simulink model "AcasXuClosedLoop_all_comp2017b"  (Simulink 9.0)
settings: Solver=VariableStepAuto  StartTime=0.0  StopTime=20  RelTol=1e-3
libraries: neural x14040, simulink x2
(part 18 of 534)

== system AcasXuClosedLoop_all_comp2017b/networks/Networks_m_4/AcasXu_2_4/Layer 3 ==
  "a{2} " : Inport  PortDimensions=50
      mask Display: e = 0.06;c1 = [120 230 180]/255;c2 = ([120 230 180]+0)/(256+128);c3 = ([120 230 180]+128)/(256+128);patch([0 1 1 0],[0 0 1 1],c1);patch([0 1 1 1-e 1-e 0+e],[0 0 1 1-e 0+e 0+e],c2);patch([0 0 1 1-e 0+e 0+e],[0 1 1 1-e 1-e 0+e],c3);plot([0 1 1 0 0],[0 0 1 1 0]);
  Delays 1 : Subsystem
      mask Display: e = 0.06;c1 = [120 150 230]/255;c2 = ([120 150 230]+0)/383;c3 = ([120 150 230]+128)/383;patch([0 1 1 0],[0 0 1 1],c1);patch([0 1 1 1-e 1-e 0+e],[0 0 1 1-e 0+e 0+e],c2);patch([0 0 1 1-e 0+e 0+e],[0 1 1 1-e 1-e 0+e],c3);plot([0 1 1 0 0],[0 0 1 1 0]);disp('0');
  "LW{3,2}" : Subsystem
      mask Display: e = 0.06;c1 = [120 150 230]/255;c2 = ([120 150 230]+0)/383;c3 = ([120 150 230]+128)/383;patch([0 1 1 0],[0 0 1 1],c1);patch([0 1 1 1-e 1-e 0+e],[0 0 1 1-e 0+e 0+e],c2);patch([0 0 1 1-e 0+e 0+e],[0 1 1 1-e 1-e 0+e],c3);plot([0 1 1 0 0],[0 0 1 1 0]);disp('W');
  "b{3}" : Constant  Value=[-0.56579800000000002313527147634886205196380615234375;0.6863989999999999813695694683701731264591217041015625;0.32173499999999999321431687349104322493076324462890625;0.07862260000000000081943341001533553935587406158447265625;-0.19624099999999999877076106713502667844295501708984375;0.122257000000000004558131649901042692363262176513671875;-1.1890300000000000313349346470204181969165802001953125;-0.8600090000000000234336994253681041300296783447265625;-1.40829000000000004177991286269389092922210693359375;-2.094539999999999846380660528666339814662933349609375;-1.008070000000000021600499167107045650482177734375;-0.342619999999999980122566967111197300255298614501953125;-0.2805210000000000203357330974540673196315765380859375;0.1626039999999999985380583211735938675701618194580078125;-1.042990000000000083701934272539801895618438720703125;0.62873400000000001508482228018692694604396820068359375;0.50152900000000000257927013080916367471218109130859375;-0.60730899999999998772892695342306979000568389892578125;-3.88682000000000016370904631912708282470703125;-0.365016999999999980364151497269631363451480865478515625;-0.251825000000000021049828546892968006432056427001953125;0.2107259999999999966480146440517273731529712677001953125;-0.99212800000000000988364945442299358546733856201171875;-0.297250999999999987455368000155431218445301055908203125;0.380684999999999995612398606681381352245807647705078125;-0.8203549999999999453592636200482957065105438232421875;-0.034085999999999998244515353462702478282153606414794921875;-0.9310359999999999747188894616556353867053985595703125;-1.346950000000000091660012913052923977375030517578125;1.1735800000000000675726141707855276763439178466796875;0.55995399999999995177546452396200038492679595947265625;-0.1108179999999999998383515276145772077143192291259765625;1.505549999999999943867123874952085316181182861328125;-0.246101999999999987434051718082628212869167327880859375;-0.72938099999999994604849007373559288680553436279296875;-1.30217999999999989313437254168093204498291015625;-0.2433309999999999917452697673070360906422138214111328125;0.790510999999999963705477057374082505702972412109375;1.1992799999999999016608853708021342754364013671875;-0.63741700000000001136157834480400197207927703857421875;-0.016549899999999999333777367382936063222587108612060546875;-0.60409900000000005260147872832021676003932952880859375;-0.46064899999999997515232053046929650008678436279296875;1.1716200000000001057998133546789176762104034423828125;0.7013759999999999994457766661071218550205230712890625;-0.18702099999999999280220208675018511712551116943359375;-0.020953800000000001590994003208834328688681125640869140625;-0.885156999999999971606712279026396572589874267578125;0.501260999999999956600049699773080646991729736328125;-1.2725400000000000044764192352886311709880828857421875]
      mask Display: e = 0.06;c1 = [120 150 230]/255;c2 = ([120 150 230]+0)/383;c3 = ([120 150 230]+128)/383;patch([0 1 1 0],[0 0 1 1],c1);patch([0 1 1 1-e 1-e 0+e],[0 0 1 1-e 0+e 0+e],c2);patch([0 0 1 1-e 0+e 0+e],[0 1 1 1-e 1-e 0+e],c3);plot([0 1 1 0 0],[0 0 1 1 0]);disp('b');
  netsum : Sum  Inputs=2  SaturateOnIntegerOverflow=on  InputSameDT=on  OutDataTypeStr=Inherit: Same as first input
      mask Display: e = 0.06;c1 = [120 150 230]/255;c2 = ([120 150 230]+0)/383;c3 = ([120 150 230]+128)/383;patch([0 1 1 0],[0 0 1 1],c1);patch([0 1 1 1-e 1-e 0+e],[0 0 1 1-e 0+e 0+e],c2);patch([0 0 1 1-e 0+e 0+e],[0 1 1 1-e 1-e 0+e],c3);plot([0 1 1 0 0],[0 0 1 1 0]);disp('+');
  poslin : neural/Transfer Functions/poslin
      settings: SourceProductName=Deep Learning Toolbox  SourceProductBaseCode=NN
  "a{3}" : Outport  InitialOutput=[0;0;0;0;0;0;0;0;0;0;0;0;0;0;0;0;0;0;0;0;0;0;0;0;0;0;0;0;0;0;0;0;0;0;0;0;0;0;0;0;0;0;0;0;0;0;0;0;0;0]
      mask Display: e = 0.06;c1 = [120 230 180]/255;c2 = ([120 230 180]+0)/(256+128);c3 = ([120 230 180]+128)/(256+128);patch([0 1 1 0],[0 0 1 1],c1);patch([0 1 1 1-e 1-e 0+e],[0 0 1 1-e 0+e 0+e],c2);patch([0 0 1 1-e 0+e 0+e],[0 1 1 1-e 1-e 0+e],c3);plot([0 1 1 0 0],[0 0 1 1 0]);
  wires:
  poslin/1 -> "a{3}"/1
  netsum/1 -> poslin/1
  "b{3}"/1 -> netsum/2
  "LW{3,2}"/1("lz{3,2}") -> netsum/1
  Delays 1/1("pd{3,2}") -> "LW{3,2}"/1("ad{3,2}")
  "a{2} "/1 -> Delays 1/1("p{3}")

== system AcasXuClosedLoop_all_comp2017b/networks/Networks_m_4/AcasXu_2_4/Layer 4 ==
  "a{3} " : Inport  PortDimensions=50
      mask Display: e = 0.06;c1 = [120 230 180]/255;c2 = ([120 230 180]+0)/(256+128);c3 = ([120 230 180]+128)/(256+128);patch([0 1 1 0],[0 0 1 1],c1);patch([0 1 1 1-e 1-e 0+e],[0 0 1 1-e 0+e 0+e],c2);patch([0 0 1 1-e 0+e 0+e],[0 1 1 1-e 1-e 0+e],c3);plot([0 1 1 0 0],[0 0 1 1 0]);
  Delays 1 : Subsystem
      mask Display: e = 0.06;c1 = [120 150 230]/255;c2 = ([120 150 230]+0)/383;c3 = ([120 150 230]+128)/383;patch([0 1 1 0],[0 0 1 1],c1);patch([0 1 1 1-e 1-e 0+e],[0 0 1 1-e 0+e 0+e],c2);patch([0 0 1 1-e 0+e 0+e],[0 1 1 1-e 1-e 0+e],c3);plot([0 1 1 0 0],[0 0 1 1 0]);disp('0');
  "LW{4,3}" : Subsystem
      mask Display: e = 0.06;c1 = [120 150 230]/255;c2 = ([120 150 230]+0)/383;c3 = ([120 150 230]+128)/383;patch([0 1 1 0],[0 0 1 1],c1);patch([0 1 1 1-e 1-e 0+e],[0 0 1 1-e 0+e 0+e],c2);patch([0 0 1 1-e 0+e 0+e],[0 1 1 1-e 1-e 0+e],c3);plot([0 1 1 0 0],[0 0 1 1 0]);disp('W');
  "b{4}" : Constant  Value=[-0.0139097000000000005359712673680405714549124240875244140625;-0.07528880000000000294324564720227499492466449737548828125;2.075530000000000097060137704829685389995574951171875;0.100026000000000003797850922637735493481159210205078125;-0.69344799999999995332444768791901879012584686279296875;-1.270750000000000046185277824406512081623077392578125;-1.3205199999999999160849029067321680486202239990234375;-1.0355399999999999050004362288746051490306854248046875;-1.220310000000000005826450433232821524143218994140625;-1.7545900000000000940048039410612545907497406005859375;-0.12118299999999999905231362617996637709438800811767578125;-2.16260999999999992127186487778089940547943115234375;-0.14306900000000000172661884789704345166683197021484375;-0.0989629999999999954152229975079535506665706634521484375;0.61942799999999997861976908097858540713787078857421875;-2.328440000000000065227823142777197062969207763671875;-0.935335999999999945231365927611477673053741455078125;-0.56300300000000003119993152722599916160106658935546875;-3.3324400000000000687805368215776979923248291015625;-1.3442600000000000104449782156734727323055267333984375;-0.0438336000000000003407052417969680391252040863037109375;0.54565099999999999713651277488679625093936920166015625;-1.4457299999999999595701183352502994239330291748046875;-0.284542000000000017134738072854815982282161712646484375;-0.56924200000000002575006874394603073596954345703125;-0.5276710000000000011510792319313623011112213134765625;0.331886999999999987576160265234648250043392181396484375;-1.167240000000000055280224842135794460773468017578125;0.59963500000000002909672502937610261142253875732421875;-1.1537599999999998967581404940574429929256439208984375;0.67512899999999997913135985072585754096508026123046875;0.046315399999999999625099889044577139429748058319091796875;-0.7551639999999999464108668689732439815998077392578125;0.90475099999999997191224565540323965251445770263671875;-1.098360000000000002984279490192420780658721923828125;0.490709000000000006291855925155687145888805389404296875;-1.830340000000000078017592386459000408649444580078125;-0.330212000000000005517364343177177943289279937744140625;-0.76566400000000001124789150708238594233989715576171875;1.3308999999999999719335619374760426580905914306640625;-0.0499606999999999967787545074315858073532581329345703125;-0.033070599999999998608313234171873773448169231414794921875;0.943799999999999972288833305356092751026153564453125;-1.420619999999999993889332472463138401508331298828125;1.1566799999999999304378661690861918032169342041015625;0.4695300000000000029132252166164107620716094970703125;-0.12093800000000000383071352416664012707769870758056640625;-1.2574799999999999311484089048462919890880584716796875;0.2654279999999999972715158946812152862548828125;-1.13037000000000009691802915767766535282135009765625]
      mask Display: e = 0.06;c1 = [120 150 230]/255;c2 = ([120 150 230]+0)/383;c3 = ([120 150 230]+128)/383;patch([0 1 1 0],[0 0 1 1],c1);patch([0 1 1 1-e 1-e 0+e],[0 0 1 1-e 0+e 0+e],c2);patch([0 0 1 1-e 0+e 0+e],[0 1 1 1-e 1-e 0+e],c3);plot([0 1 1 0 0],[0 0 1 1 0]);disp('b');
  netsum : Sum  Inputs=2  SaturateOnIntegerOverflow=on  InputSameDT=on  OutDataTypeStr=Inherit: Same as first input
      mask Display: e = 0.06;c1 = [120 150 230]/255;c2 = ([120 150 230]+0)/383;c3 = ([120 150 230]+128)/383;patch([0 1 1 0],[0 0 1 1],c1);patch([0 1 1 1-e 1-e 0+e],[0 0 1 1-e 0+e 0+e],c2);patch([0 0 1 1-e 0+e 0+e],[0 1 1 1-e 1-e 0+e],c3);plot([0 1 1 0 0],[0 0 1 1 0]);disp('+');
  poslin : neural/Transfer Functions/poslin
      settings: SourceProductName=Deep Learning Toolbox  SourceProductBaseCode=NN
  "a{4}" : Outport  InitialOutput=[0;0;0;0;0;0;0;0;0;0;0;0;0;0;0;0;0;0;0;0;0;0;0;0;0;0;0;0;0;0;0;0;0;0;0;0;0;0;0;0;0;0;0;0;0;0;0;0;0;0]
      mask Display: e = 0.06;c1 = [120 230 180]/255;c2 = ([120 230 180]+0)/(256+128);c3 = ([120 230 180]+128)/(256+128);patch([0 1 1 0],[0 0 1 1],c1);patch([0 1 1 1-e 1-e 0+e],[0 0 1 1-e 0+e 0+e],c2);patch([0 0 1 1-e 0+e 0+e],[0 1 1 1-e 1-e 0+e],c3);plot([0 1 1 0 0],[0 0 1 1 0]);
  wires:
  poslin/1 -> "a{4}"/1
  netsum/1 -> poslin/1
  "b{4}"/1 -> netsum/2
  "LW{4,3}"/1("lz{4,3}") -> netsum/1
  Delays 1/1("pd{4,3}") -> "LW{4,3}"/1("ad{4,3}")
  "a{3} "/1 -> Delays 1/1("p{4}")

== system AcasXuClosedLoop_all_comp2017b/networks/Networks_m_4/AcasXu_2_4/Layer 5 ==
  "a{4} " : Inport  PortDimensions=50
      mask Display: e = 0.06;c1 = [120 230 180]/255;c2 = ([120 230 180]+0)/(256+128);c3 = ([120 230 180]+128)/(256+128);patch([0 1 1 0],[0 0 1 1],c1);patch([0 1 1 1-e 1-e 0+e],[0 0 1 1-e 0+e 0+e],c2);patch([0 0 1 1-e 0+e 0+e],[0 1 1 1-e 1-e 0+e],c3);plot([0 1 1 0 0],[0 0 1 1 0]);
  Delays 1 : Subsystem
      mask Display: e = 0.06;c1 = [120 150 230]/255;c2 = ([120 150 230]+0)/383;c3 = ([120 150 230]+128)/383;patch([0 1 1 0],[0 0 1 1],c1);patch([0 1 1 1-e 1-e 0+e],[0 0 1 1-e 0+e 0+e],c2);patch([0 0 1 1-e 0+e 0+e],[0 1 1 1-e 1-e 0+e],c3);plot([0 1 1 0 0],[0 0 1 1 0]);disp('0');
  "LW{5,4}" : Subsystem
      mask Display: e = 0.06;c1 = [120 150 230]/255;c2 = ([120 150 230]+0)/383;c3 = ([120 150 230]+128)/383;patch([0 1 1 0],[0 0 1 1],c1);patch([0 1 1 1-e 1-e 0+e],[0 0 1 1-e 0+e 0+e],c2);patch([0 0 1 1-e 0+e 0+e],[0 1 1 1-e 1-e 0+e],c3);plot([0 1 1 0 0],[0 0 1 1 0]);disp('W');
  "b{5}" : Constant  Value=[-0.10940999999999999336974809693856514059007167816162109375;-4.41561000000000003495870259939692914485931396484375;1.2665800000000000391509047403815202414989471435546875;-0.457340000000000024282797994601423852145671844482421875;2.1883499999999997953636921010911464691162109375;-0.366215000000000012736478538499795831739902496337890625;0.365192000000000016601831021034740842878818511962890625;-1.48285000000000000142108547152020037174224853515625;0.1785130000000000050075499302693060599267482757568359375;0.09404089999999999671675965373651706613600254058837890625;0.5114760000000000417230694438330829143524169921875;-1.9412700000000000510880227011512033641338348388671875;-0.70229799999999997783817207164247520267963409423828125;-0.141270000000000006679101716144941747188568115234375;1.062689999999999912461134954355657100677490234375;0.52761899999999994914645640164962969720363616943359375;0.78126799999999996249044897922431118786334991455078125;-1.9535199999999999231903302643331699073314666748046875;-2.3968199999999999505462255910970270633697509765625;-0.78913599999999994860644392247195355594158172607421875;0.2007210000000000105213615597676835022866725921630859375;-0.77595599999999997908872728658025152981281280517578125;0.717330000000000023163693185779266059398651123046875;-1.6638800000000000256505927609396167099475860595703125;1.08101000000000002643218977027572691440582275390625;-0.0638539999999999940971662226729677058756351470947265625;1.567190000000000082991391536779701709747314453125;0.041038499999999998590904937145751318894326686859130859375;1.7739799999999998902922015986405313014984130859375;-0.0660338000000000036049385698788682930171489715576171875;0.495254000000000027537083724382682703435420989990234375;-2.619190000000000129176669361186213791370391845703125;1.66504999999999991899812812334857881069183349609375;-0.67660299999999995446131606513517908751964569091796875;0.41489100000000000978417347141657955944538116455078125;-1.2167600000000000637356833976809866726398468017578125;1.1150599999999999401012473754235543310642242431640625;0.9283310000000000172803993336856365203857421875;0.2296999999999999875210932032132404856383800506591796875;0.053061999999999998056665617696125991642475128173828125;2.1252300000000001745092959026806056499481201171875;-1.03211999999999992638777257525362074375152587890625;0.6291889999999999982804865794605575501918792724609375;-3.50731999999999999317878973670303821563720703125;-0.507355000000000000426325641456060111522674560546875;0.372373000000000009546141654936945997178554534912109375;0.9320180000000000131166189021314494311809539794921875;-0.50340200000000001612221467439667321741580963134765625;-1.4255400000000000293454149868921376764774322509765625;0.883040999999999964842345434590242803096771240234375]
      mask Display: e = 0.06;c1 = [120 150 230]/255;c2 = ([120 150 230]+0)/383;c3 = ([120 150 230]+128)/383;patch([0 1 1 0],[0 0 1 1],c1);patch([0 1 1 1-e 1-e 0+e],[0 0 1 1-e 0+e 0+e],c2);patch([0 0 1 1-e 0+e 0+e],[0 1 1 1-e 1-e 0+e],c3);plot([0 1 1 0 0],[0 0 1 1 0]);disp('b');
  netsum : Sum  Inputs=2  SaturateOnIntegerOverflow=on  InputSameDT=on  OutDataTypeStr=Inherit: Same as first input
      mask Display: e = 0.06;c1 = [120 150 230]/255;c2 = ([120 150 230]+0)/383;c3 = ([120 150 230]+128)/383;patch([0 1 1 0],[0 0 1 1],c1);patch([0 1 1 1-e 1-e 0+e],[0 0 1 1-e 0+e 0+e],c2);patch([0 0 1 1-e 0+e 0+e],[0 1 1 1-e 1-e 0+e],c3);plot([0 1 1 0 0],[0 0 1 1 0]);disp('+');
  poslin : neural/Transfer Functions/poslin
      settings: SourceProductName=Deep Learning Toolbox  SourceProductBaseCode=NN
  "a{5}" : Outport  InitialOutput=[0;0;0;0;0;0;0;0;0;0;0;0;0;0;0;0;0;0;0;0;0;0;0;0;0;0;0;0;0;0;0;0;0;0;0;0;0;0;0;0;0;0;0;0;0;0;0;0;0;0]
      mask Display: e = 0.06;c1 = [120 230 180]/255;c2 = ([120 230 180]+0)/(256+128);c3 = ([120 230 180]+128)/(256+128);patch([0 1 1 0],[0 0 1 1],c1);patch([0 1 1 1-e 1-e 0+e],[0 0 1 1-e 0+e 0+e],c2);patch([0 0 1 1-e 0+e 0+e],[0 1 1 1-e 1-e 0+e],c3);plot([0 1 1 0 0],[0 0 1 1 0]);
  wires:
  poslin/1 -> "a{5}"/1
  netsum/1 -> poslin/1
  "b{5}"/1 -> netsum/2
  "LW{5,4}"/1("lz{5,4}") -> netsum/1
  Delays 1/1("pd{5,4}") -> "LW{5,4}"/1("ad{5,4}")
  "a{4} "/1 -> Delays 1/1("p{5}")

== system AcasXuClosedLoop_all_comp2017b/networks/Networks_m_4/AcasXu_2_4/Layer 6 ==
  "a{5} " : Inport  PortDimensions=50
      mask Display: e = 0.06;c1 = [120 230 180]/255;c2 = ([120 230 180]+0)/(256+128);c3 = ([120 230 180]+128)/(256+128);patch([0 1 1 0],[0 0 1 1],c1);patch([0 1 1 1-e 1-e 0+e],[0 0 1 1-e 0+e 0+e],c2);patch([0 0 1 1-e 0+e 0+e],[0 1 1 1-e 1-e 0+e],c3);plot([0 1 1 0 0],[0 0 1 1 0]);
  Delays 1 : Subsystem
      mask Display: e = 0.06;c1 = [120 150 230]/255;c2 = ([120 150 230]+0)/383;c3 = ([120 150 230]+128)/383;patch([0 1 1 0],[0 0 1 1],c1);patch([0 1 1 1-e 1-e 0+e],[0 0 1 1-e 0+e 0+e],c2);patch([0 0 1 1-e 0+e 0+e],[0 1 1 1-e 1-e 0+e],c3);plot([0 1 1 0 0],[0 0 1 1 0]);disp('0');
  "LW{6,5}" : Subsystem
      mask Display: e = 0.06;c1 = [120 150 230]/255;c2 = ([120 150 230]+0)/383;c3 = ([120 150 230]+128)/383;patch([0 1 1 0],[0 0 1 1],c1);patch([0 1 1 1-e 1-e 0+e],[0 0 1 1-e 0+e 0+e],c2);patch([0 0 1 1-e 0+e 0+e],[0 1 1 1-e 1-e 0+e],c3);plot([0 1 1 0 0],[0 0 1 1 0]);disp('W');
  "b{6}" : Constant  Value=[0.2346999999999999919619853017138666473329067230224609375;-1.9369099999999999095479097377392463386058807373046875;-0.475692999999999976967757220336352474987506866455078125;-0.384604000000000001424638185199000872671604156494140625;-0.023999300000000001187316911455127410590648651123046875;0.126871000000000011542766742422827519476413726806640625;0.132994000000000001104893954106955789029598236083984375;-1.081090000000000106439301816863007843494415283203125;0.060183500000000000884625706021324731409549713134765625;-0.154803999999999997161381770638399757444858551025390625;-0.71192200000000005477573949974612332880496978759765625;-0.60855899999999996108357436241931281983852386474609375;0.05336849999999999927258187426559743471443653106689453125;0.055166500000000000258904009342586505226790904998779296875;0.449330999999999980420994916130439378321170806884765625;-0.33911999999999997701394249816075898706912994384765625;-0.0885939000000000032031266528065316379070281982421875;-2.646549999999999958077978590154089033603668212890625;-0.194010999999999989018562018827651627361774444580078125;0.414953999999999989523047361217322759330272674560546875;0.492707999999999979312548248344683088362216949462890625;-0.10349999999999999478195178426176426000893115997314453125;0.38083299999999997709210219909437000751495361328125;0.09757749999999999757083202212015748955309391021728515625;-0.315176999999999984947152142922277562320232391357421875;0.301354999999999983995024877003743313252925872802734375;-0.9592460000000000430731006417772732675075531005859375;-0.4030110000000000081143980423803441226482391357421875;-0.2432850000000000012523315717771765775978565216064453125;0.464538999999999979717557607727940194308757781982421875;0.244925000000000003819167204710538499057292938232421875;-0.6850840000000000262758703684085048735141754150390625;-0.34977999999999997982058630441315472126007080078125;-0.8555509999999999504183278986602090299129486083984375;-2.034009999999999873665501581854186952114105224609375;-0.36635200000000001097077984013594686985015869140625;0.014788400000000000156230584025252028368413448333740234375;0.319072999999999995512922623674967326223850250244140625;0.426536999999999999477751089216326363384723663330078125;0.262087000000000014399148540178430266678333282470703125;-0.1453480000000000049720227934813010506331920623779296875;-0.7604319999999999968309793985099531710147857666015625;-2.73768000000000011340262062731198966503143310546875;0.113178000000000000824229573481716215610504150390625;-0.054530900000000000205080397108758916147053241729736328125;0.1973979999999999901394431844892096705734729766845703125;-3.407529999999999947846163195208646357059478759765625;-0.05762799999999999867039690570891252718865871429443359375;-0.7910120000000000484163820146932266652584075927734375;0.7156569999999999875939238336286507546901702880859375]
      mask Display: e = 0.06;c1 = [120 150 230]/255;c2 = ([120 150 230]+0)/383;c3 = ([120 150 230]+128)/383;patch([0 1 1 0],[0 0 1 1],c1);patch([0 1 1 1-e 1-e 0+e],[0 0 1 1-e 0+e 0+e],c2);patch([0 0 1 1-e 0+e 0+e],[0 1 1 1-e 1-e 0+e],c3);plot([0 1 1 0 0],[0 0 1 1 0]);disp('b');
  netsum : Sum  Inputs=2  SaturateOnIntegerOverflow=on  InputSameDT=on  OutDataTypeStr=Inherit: Same as first input
      mask Display: e = 0.06;c1 = [120 150 230]/255;c2 = ([120 150 230]+0)/383;c3 = ([120 150 230]+128)/383;patch([0 1 1 0],[0 0 1 1],c1);patch([0 1 1 1-e 1-e 0+e],[0 0 1 1-e 0+e 0+e],c2);patch([0 0 1 1-e 0+e 0+e],[0 1 1 1-e 1-e 0+e],c3);plot([0 1 1 0 0],[0 0 1 1 0]);disp('+');
  poslin : neural/Transfer Functions/poslin
      settings: SourceProductName=Deep Learning Toolbox  SourceProductBaseCode=NN
  "a{6}" : Outport  InitialOutput=[0;0;0;0;0;0;0;0;0;0;0;0;0;0;0;0;0;0;0;0;0;0;0;0;0;0;0;0;0;0;0;0;0;0;0;0;0;0;0;0;0;0;0;0;0;0;0;0;0;0]
      mask Display: e = 0.06;c1 = [120 230 180]/255;c2 = ([120 230 180]+0)/(256+128);c3 = ([120 230 180]+128)/(256+128);patch([0 1 1 0],[0 0 1 1],c1);patch([0 1 1 1-e 1-e 0+e],[0 0 1 1-e 0+e 0+e],c2);patch([0 0 1 1-e 0+e 0+e],[0 1 1 1-e 1-e 0+e],c3);plot([0 1 1 0 0],[0 0 1 1 0]);
  wires:
  poslin/1 -> "a{6}"/1
  netsum/1 -> poslin/1
  "b{6}"/1 -> netsum/2
  "LW{6,5}"/1("lz{6,5}") -> netsum/1
  Delays 1/1("pd{6,5}") -> "LW{6,5}"/1("ad{6,5}")
  "a{5} "/1 -> Delays 1/1("p{6}")

== system AcasXuClosedLoop_all_comp2017b/networks/Networks_m_4/AcasXu_2_4/Layer 7 ==
  "a{6} " : Inport  PortDimensions=50
      mask Display: e = 0.06;c1 = [120 230 180]/255;c2 = ([120 230 180]+0)/(256+128);c3 = ([120 230 180]+128)/(256+128);patch([0 1 1 0],[0 0 1 1],c1);patch([0 1 1 1-e 1-e 0+e],[0 0 1 1-e 0+e 0+e],c2);patch([0 0 1 1-e 0+e 0+e],[0 1 1 1-e 1-e 0+e],c3);plot([0 1 1 0 0],[0 0 1 1 0]);
  Delays 1 : Subsystem
      mask Display: e = 0.06;c1 = [120 150 230]/255;c2 = ([120 150 230]+0)/383;c3 = ([120 150 230]+128)/383;patch([0 1 1 0],[0 0 1 1],c1);patch([0 1 1 1-e 1-e 0+e],[0 0 1 1-e 0+e 0+e],c2);patch([0 0 1 1-e 0+e 0+e],[0 1 1 1-e 1-e 0+e],c3);plot([0 1 1 0 0],[0 0 1 1 0]);disp('0');
  "LW{7,6}" : Subsystem
      mask Display: e = 0.06;c1 = [120 150 230]/255;c2 = ([120 150 230]+0)/383;c3 = ([120 150 230]+128)/383;patch([0 1 1 0],[0 0 1 1],c1);patch([0 1 1 1-e 1-e 0+e],[0 0 1 1-e 0+e 0+e],c2);patch([0 0 1 1-e 0+e 0+e],[0 1 1 1-e 1-e 0+e],c3);plot([0 1 1 0 0],[0 0 1 1 0]);disp('W');
  "b{7}" : Constant  Value=[-0.0198037999999999998756994301629674737341701984405517578125;-0.0203166000000000006309175404339839587919414043426513671875;0.0185957999999999991358468065527631551958620548248291015625;-0.01765529999999999877235978829048690386116504669189453125;0.0188563999999999988566035113990437821485102176666259765625]
      mask Display: e = 0.06;c1 = [120 150 230]/255;c2 = ([120 150 230]+0)/383;c3 = ([120 150 230]+128)/383;patch([0 1 1 0],[0 0 1 1],c1);patch([0 1 1 1-e 1-e 0+e],[0 0 1 1-e 0+e 0+e],c2);patch([0 0 1 1-e 0+e 0+e],[0 1 1 1-e 1-e 0+e],c3);plot([0 1 1 0 0],[0 0 1 1 0]);disp('b');
  netsum : Sum  Inputs=2  SaturateOnIntegerOverflow=on  InputSameDT=on  OutDataTypeStr=Inherit: Same as first input
      mask Display: e = 0.06;c1 = [120 150 230]/255;c2 = ([120 150 230]+0)/383;c3 = ([120 150 230]+128)/383;patch([0 1 1 0],[0 0 1 1],c1);patch([0 1 1 1-e 1-e 0+e],[0 0 1 1-e 0+e 0+e],c2);patch([0 0 1 1-e 0+e 0+e],[0 1 1 1-e 1-e 0+e],c3);plot([0 1 1 0 0],[0 0 1 1 0]);disp('+');
  purelin : neural/Transfer Functions/purelin
      settings: SourceProductName=Deep Learning Toolbox
  "a{7}" : Outport  InitialOutput=[0;0;0;0;0]
      mask Display: e = 0.06;c1 = [120 230 180]/255;c2 = ([120 230 180]+0)/(256+128);c3 = ([120 230 180]+128)/(256+128);patch([0 1 1 0],[0 0 1 1],c1);patch([0 1 1 1-e 1-e 0+e],[0 0 1 1-e 0+e 0+e],c2);patch([0 0 1 1-e 0+e 0+e],[0 1 1 1-e 1-e 0+e],c3);plot([0 1 1 0 0],[0 0 1 1 0]);
  wires:
  purelin/1 -> "a{7}"/1
  netsum/1 -> purelin/1
  "b{7}"/1 -> netsum/2
  "LW{7,6}"/1("lz{7,6}") -> netsum/1
  Delays 1/1("pd{7,6}") -> "LW{7,6}"/1("ad{7,6}")
  "a{6} "/1 -> Delays 1/1("p{7}")

== system AcasXuClosedLoop_all_comp2017b/networks/Networks_m_4/AcasXu_2_4/Process Input 1 ==
  x : Inport  PortDimensions=5
      mask Display: e = 0.06;c1 = [120 230 180]/255;c2 = ([120 230 180]+0)/(256+128);c3 = ([120 230 180]+128)/(256+128);patch([0 1 1 0],[0 0 1 1],c1);patch([0 1 1 1-e 1-e 0+e],[0 0 1 1-e 0+e 0+e],c2);patch([0 0 1 1-e 0+e 0+e],[0 1 1 1-e 1-e 0+e],c3);plot([0 1 1 0 0],[0 0 1 1 0]);
  p : Outport  PortDimensions=5
      mask Display: e = 0.06;c1 = [120 230 180]/255;c2 = ([120 230 180]+0)/(256+128);c3 = ([120 230 180]+128)/(256+128);patch([0 1 1 0],[0 0 1 1],c1);patch([0 1 1 1-e 1-e 0+e],[0 0 1 1-e 0+e 0+e],c2);patch([0 0 1 1-e 0+e 0+e],[0 1 1 1-e 1-e 0+e],c3);plot([0 1 1 0 0],[0 0 1 1 0]);
  wires:
  x/1 -> p/1

== system AcasXuClosedLoop_all_comp2017b/networks/Networks_m_4/AcasXu_2_4/Process Output 1 ==
  a : Inport  PortDimensions=5
      mask Display: e = 0.06;c1 = [120 230 180]/255;c2 = ([120 230 180]+0)/(256+128);c3 = ([120 230 180]+128)/(256+128);patch([0 1 1 0],[0 0 1 1],c1);patch([0 1 1 1-e 1-e 0+e],[0 0 1 1-e 0+e 0+e],c2);patch([0 0 1 1-e 0+e 0+e],[0 1 1 1-e 1-e 0+e],c3);plot([0 1 1 0 0],[0 0 1 1 0]);
  y : Outport  PortDimensions=5
      mask Display: e = 0.06;c1 = [120 230 180]/255;c2 = ([120 230 180]+0)/(256+128);c3 = ([120 230 180]+128)/(256+128);patch([0 1 1 0],[0 0 1 1],c1);patch([0 1 1 1-e 1-e 0+e],[0 0 1 1-e 0+e 0+e],c2);patch([0 0 1 1-e 0+e 0+e],[0 1 1 1-e 1-e 0+e],c3);plot([0 1 1 0 0],[0 0 1 1 0]);
  wires:
  a/1 -> y/1

== system AcasXuClosedLoop_all_comp2017b/networks/Networks_m_4/AcasXu_3_4/Layer 1 ==
  "p{1}" : Inport  PortDimensions=5
      mask Display: e = 0.06;c1 = [120 230 180]/255;c2 = ([120 230 180]+0)/(256+128);c3 = ([120 230 180]+128)/(256+128);patch([0 1 1 0],[0 0 1 1],c1);patch([0 1 1 1-e 1-e 0+e],[0 0 1 1-e 0+e 0+e],c2);patch([0 0 1 1-e 0+e 0+e],[0 1 1 1-e 1-e 0+e],c3);plot([0 1 1 0 0],[0 0 1 1 0]);
  Delays 1 : Subsystem
      mask Display: e = 0.06;c1 = [120 150 230]/255;c2 = ([120 150 230]+0)/383;c3 = ([120 150 230]+128)/383;patch([0 1 1 0],[0 0 1 1],c1);patch([0 1 1 1-e 1-e 0+e],[0 0 1 1-e 0+e 0+e],c2);patch([0 0 1 1-e 0+e 0+e],[0 1 1 1-e 1-e 0+e],c3);plot([0 1 1 0 0],[0 0 1 1 0]);disp('0');
  "IW{1,1}" : Subsystem
      mask Display: e = 0.06;c1 = [120 150 230]/255;c2 = ([120 150 230]+0)/383;c3 = ([120 150 230]+128)/383;patch([0 1 1 0],[0 0 1 1],c1);patch([0 1 1 1-e 1-e 0+e],[0 0 1 1-e 0+e 0+e],c2);patch([0 0 1 1-e 0+e 0+e],[0 1 1 1-e 1-e 0+e],c3);plot([0 1 1 0 0],[0 0 1 1 0]);disp('W');
  "b{1}" : Constant  Value=[-0.298177999999999998603783524231403134763240814208984375;-0.1520769999999999899653602142279851250350475311279296875;-0.01949789999999999856594712355217779986560344696044921875;0.164283000000000012352785461189341731369495391845703125;0.288163000000000002476241434123949147760868072509765625;-0.37496099999999998875210849291761405766010284423828125;0.224231000000000013638867812915123067796230316162109375;0.2087849999999999983657517077517695724964141845703125;-0.446647000000000016228796084760688245296478271484375;-0.01147600000000000008970602038971264846622943878173828125;-0.42160399999999997877608848284580744802951812744140625;0.0178613000000000000933031429894981556572020053863525390625;-0.350032999999999983042897611085209064185619354248046875;-0.55450399999999999689492824472836218774318695068359375;-0.057485500000000001874500554777114302851259708404541015625;0.2168529999999999902104974580652196891605854034423828125;0.256390999999999980030196411462384276092052459716796875;-0.06786899999999999877342560239412705413997173309326171875;0.03457670000000000187778681493000476621091365814208984375;0.0294957999999999991025401158140084589831531047821044921875;-0.34781899999999998929212097209529019892215728759765625;0.22080800000000000427036184191820211708545684814453125;0.1217479999999999951132423348099109716713428497314453125;-0.141120999999999996443733607520698569715023040771484375;0.72653400000000001313082975684665143489837646484375;0.047994299999999996686472769624742795713245868682861328125;0.1301170000000000104289910041188704781234264373779296875;-0.062357500000000003204103649068201775662600994110107421875;0.168513999999999997125854633850394748151302337646484375;-0.6326169999999999848938614377402700483798980712890625;0.734766000000000030212277124519459903240203857421875;0.0274738999999999990164756269450663239695131778717041015625;-0.0209650000000000009958700530887654167599976062774658203125;0.2687880000000000269011479758773930370807647705078125;0.312273999999999996024513393422239460051059722900390625;-0.1485160000000000091180396566414856351912021636962890625;-0.47923999999999999932498440102790482342243194580078125;0.2259670000000000011919354392375680617988109588623046875;0.11329799999999999593480737303252681158483028411865234375;-0.0224934000000000001995292819856331334449350833892822265625;0.208405000000000006910028105266974307596683502197265625;0.406106000000000022520652009916375391185283660888671875;0.204786999999999996813215830115950666368007659912109375;0.1929579999999999906368230995212798006832599639892578125;0.07570590000000000652224940722589963115751743316650390625;-0.35659899999999999931077354631270281970500946044921875;-0.03019940000000000124291688052835525013506412506103515625;0.051788000000000000699884594723698683083057403564453125;-0.55582399999999998474464746323064900934696197509765625;-0.08860949999999999382449544782502925954759120941162109375]
      mask Display: e = 0.06;c1 = [120 150 230]/255;c2 = ([120 150 230]+0)/383;c3 = ([120 150 230]+128)/383;patch([0 1 1 0],[0 0 1 1],c1);patch([0 1 1 1-e 1-e 0+e],[0 0 1 1-e 0+e 0+e],c2);patch([0 0 1 1-e 0+e 0+e],[0 1 1 1-e 1-e 0+e],c3);plot([0 1 1 0 0],[0 0 1 1 0]);disp('b');
  netsum : Sum  Inputs=2  SaturateOnIntegerOverflow=on  InputSameDT=on  OutDataTypeStr=Inherit: Same as first input
      mask Display: e = 0.06;c1 = [120 150 230]/255;c2 = ([120 150 230]+0)/383;c3 = ([120 150 230]+128)/383;patch([0 1 1 0],[0 0 1 1],c1);patch([0 1 1 1-e 1-e 0+e],[0 0 1 1-e 0+e 0+e],c2);patch([0 0 1 1-e 0+e 0+e],[0 1 1 1-e 1-e 0+e],c3);plot([0 1 1 0 0],[0 0 1 1 0]);disp('+');
  poslin : neural/Transfer Functions/poslin
      settings: SourceProductName=Deep Learning Toolbox  SourceProductBaseCode=NN
  "a{1}" : Outport  InitialOutput=[0;0;0;0;0;0;0;0;0;0;0;0;0;0;0;0;0;0;0;0;0;0;0;0;0;0;0;0;0;0;0;0;0;0;0;0;0;0;0;0;0;0;0;0;0;0;0;0;0;0]
      mask Display: e = 0.06;c1 = [120 230 180]/255;c2 = ([120 230 180]+0)/(256+128);c3 = ([120 230 180]+128)/(256+128);patch([0 1 1 0],[0 0 1 1],c1);patch([0 1 1 1-e 1-e 0+e],[0 0 1 1-e 0+e 0+e],c2);patch([0 0 1 1-e 0+e 0+e],[0 1 1 1-e 1-e 0+e],c3);plot([0 1 1 0 0],[0 0 1 1 0]);
  wires:
  poslin/1 -> "a{1}"/1
  netsum/1 -> poslin/1
  "b{1}"/1 -> netsum/2
  "IW{1,1}"/1("iz{1,1}") -> netsum/1
  Delays 1/1("pd{1,1}") -> "IW{1,1}"/1("pd{1,1}")
  "p{1}"/1 -> Delays 1/1("p{1}")

== system AcasXuClosedLoop_all_comp2017b/networks/Networks_m_4/AcasXu_3_4/Layer 2 ==
  "a{1} " : Inport  PortDimensions=50
      mask Display: e = 0.06;c1 = [120 230 180]/255;c2 = ([120 230 180]+0)/(256+128);c3 = ([120 230 180]+128)/(256+128);patch([0 1 1 0],[0 0 1 1],c1);patch([0 1 1 1-e 1-e 0+e],[0 0 1 1-e 0+e 0+e],c2);patch([0 0 1 1-e 0+e 0+e],[0 1 1 1-e 1-e 0+e],c3);plot([0 1 1 0 0],[0 0 1 1 0]);
  Delays 1 : Subsystem
      mask Display: e = 0.06;c1 = [120 150 230]/255;c2 = ([120 150 230]+0)/383;c3 = ([120 150 230]+128)/383;patch([0 1 1 0],[0 0 1 1],c1);patch([0 1 1 1-e 1-e 0+e],[0 0 1 1-e 0+e 0+e],c2);patch([0 0 1 1-e 0+e 0+e],[0 1 1 1-e 1-e 0+e],c3);plot([0 1 1 0 0],[0 0 1 1 0]);disp('0');
  "LW{2,1}" : Subsystem
      mask Display: e = 0.06;c1 = [120 150 230]/255;c2 = ([120 150 230]+0)/383;c3 = ([120 150 230]+128)/383;patch([0 1 1 0],[0 0 1 1],c1);patch([0 1 1 1-e 1-e 0+e],[0 0 1 1-e 0+e 0+e],c2);patch([0 0 1 1-e 0+e 0+e],[0 1 1 1-e 1-e 0+e],c3);plot([0 1 1 0 0],[0 0 1 1 0]);disp('W');
  "b{2}" : Constant  Value=[-0.12312299999999999633448766189758316613733768463134765625;-0.50260000000000004671818487622658722102642059326171875;-0.1524040000000000116830989327354473061859607696533203125;-0.5761629999999999807158701514708809554576873779296875;-0.1137100000000000055155879863377776928246021270751953125;0.172109000000000011976197811236488632857799530029296875;-0.01337559999999999964248598161020709085278213024139404296875;-0.64465099999999997404387386268354021012783050537109375;-0.00993046000000000032847946584979581530205905437469482421875;0.21597399999999999931077354631270281970500946044921875;0;-0.464461999999999985977439109774422831833362579345703125;-0.4217210000000000125197630040929652750492095947265625;-0.6042389999999999705693198848166503012180328369140625;-0.23877599999999998825472857788554392755031585693359375;-0.67432199999999997697130993401515297591686248779296875;-0.57196499999999994567900785114034079015254974365234375;-0.00859571999999999940278438970153729314915835857391357421875;-0.27465200000000000724043047739542089402675628662109375;-0.63266100000000002889777306336327455937862396240234375;0.6497770000000000489848162033013068139553070068359375;0.72945599999999999329958200178225524723529815673828125;0.5844409999999999882902557146735489368438720703125;0.281538000000000010469847211425076238811016082763671875;-0.01352339999999999965718533445624416344799101352691650390625;0.3863929999999999864712663111276924610137939453125;0.00093084299999999999160327224245747856912203133106231689453125;0.0976679000000000019365842263141530565917491912841796875;-0.7310309999999999863717903281212784349918365478515625;-0.08011859999999999815134543723615934140980243682861328125;-0.48816300000000001357847168037551455199718475341796875;0.315908999999999995367261362844146788120269775390625;-0.01777120000000000088480334170526475645601749420166015625;0.407102000000000019408474827287136577069759368896484375;-0.50529900000000005366729283196036703884601593017578125;-0.69190899999999999625543978254427202045917510986328125;-0.00915854999999999967730257566245199996046721935272216796875;-0.0176381000000000003058442388237381237559020519256591796875;0.183715999999999990421883921953849494457244873046875;-0.004622560000000000383912901469329881365410983562469482421875;-1.5539899999999999824495944267255254089832305908203125;0.166302000000000005375255796025157906115055084228515625;-0.50364500000000000934363697524531744420528411865234375;-1.0394600000000000505906427861191332340240478515625;1.012539999999999995594635038287378847599029541015625;-0.3882309999999999927666749499621801078319549560546875;-0.366972000000000020403234657351276837289333343505859375;-0.79486800000000001897859647215227596461772918701171875;-0.0154842000000000000470290473231216310523450374603271484375;0.490416000000000018577139826447819359600543975830078125]
      mask Display: e = 0.06;c1 = [120 150 230]/255;c2 = ([120 150 230]+0)/383;c3 = ([120 150 230]+128)/383;patch([0 1 1 0],[0 0 1 1],c1);patch([0 1 1 1-e 1-e 0+e],[0 0 1 1-e 0+e 0+e],c2);patch([0 0 1 1-e 0+e 0+e],[0 1 1 1-e 1-e 0+e],c3);plot([0 1 1 0 0],[0 0 1 1 0]);disp('b');
  netsum : Sum  Inputs=2  SaturateOnIntegerOverflow=on  InputSameDT=on  OutDataTypeStr=Inherit: Same as first input
      mask Display: e = 0.06;c1 = [120 150 230]/255;c2 = ([120 150 230]+0)/383;c3 = ([120 150 230]+128)/383;patch([0 1 1 0],[0 0 1 1],c1);patch([0 1 1 1-e 1-e 0+e],[0 0 1 1-e 0+e 0+e],c2);patch([0 0 1 1-e 0+e 0+e],[0 1 1 1-e 1-e 0+e],c3);plot([0 1 1 0 0],[0 0 1 1 0]);disp('+');
  poslin : neural/Transfer Functions/poslin
      settings: SourceProductName=Deep Learning Toolbox  SourceProductBaseCode=NN
  "a{2}" : Outport  InitialOutput=[0;0;0;0;0;0;0;0;0;0;0;0;0;0;0;0;0;0;0;0;0;0;0;0;0;0;0;0;0;0;0;0;0;0;0;0;0;0;0;0;0;0;0;0;0;0;0;0;0;0]
      mask Display: e = 0.06;c1 = [120 230 180]/255;c2 = ([120 230 180]+0)/(256+128);c3 = ([120 230 180]+128)/(256+128);patch([0 1 1 0],[0 0 1 1],c1);patch([0 1 1 1-e 1-e 0+e],[0 0 1 1-e 0+e 0+e],c2);patch([0 0 1 1-e 0+e 0+e],[0 1 1 1-e 1-e 0+e],c3);plot([0 1 1 0 0],[0 0 1 1 0]);
  wires:
  poslin/1 -> "a{2}"/1
  netsum/1 -> poslin/1
  "b{2}"/1 -> netsum/2
  "LW{2,1}"/1("lz{2,1}") -> netsum/1
  Delays 1/1("pd{2,1}") -> "LW{2,1}"/1("ad{2,1}")
  "a{1} "/1 -> Delays 1/1("p{2}")

== system AcasXuClosedLoop_all_comp2017b/networks/Networks_m_4/AcasXu_3_4/Layer 3 ==
  "a{2} " : Inport  PortDimensions=50
      mask Display: e = 0.06;c1 = [120 230 180]/255;c2 = ([120 230 180]+0)/(256+128);c3 = ([120 230 180]+128)/(256+128);patch([0 1 1 0],[0 0 1 1],c1);patch([0 1 1 1-e 1-e 0+e],[0 0 1 1-e 0+e 0+e],c2);patch([0 0 1 1-e 0+e 0+e],[0 1 1 1-e 1-e 0+e],c3);plot([0 1 1 0 0],[0 0 1 1 0]);
  Delays 1 : Subsystem
      mask Display: e = 0.06;c1 = [120 150 230]/255;c2 = ([120 150 230]+0)/383;c3 = ([120 150 230]+128)/383;patch([0 1 1 0],[0 0 1 1],c1);patch([0 1 1 1-e 1-e 0+e],[0 0 1 1-e 0+e 0+e],c2);patch([0 0 1 1-e 0+e 0+e],[0 1 1 1-e 1-e 0+e],c3);plot([0 1 1 0 0],[0 0 1 1 0]);disp('0');
  "LW{3,2}" : Subsystem
      mask Display: e = 0.06;c1 = [120 150 230]/255;c2 = ([120 150 230]+0)/383;c3 = ([120 150 230]+128)/383;patch([0 1 1 0],[0 0 1 1],c1);patch([0 1 1 1-e 1-e 0+e],[0 0 1 1-e 0+e 0+e],c2);patch([0 0 1 1-e 0+e 0+e],[0 1 1 1-e 1-e 0+e],c3);plot([0 1 1 0 0],[0 0 1 1 0]);disp('W');
  "b{3}" : Constant  Value=[1.048799999999999954525264911353588104248046875;-0.0166157000000000006079137193637507152743637561798095703125;0.6152320000000000010942358130705542862415313720703125;-1.2198599999999999443645037899841554462909698486328125;-0.455618999999999996219912645756267011165618896484375;-1.287339999999999928803617876837961375713348388671875;-1.590030000000000054427573559223674237728118896484375;-0.385855000000000003534950110406498424708843231201171875;1.09769000000000005456968210637569427490234375;0.313655999999999990368593216771841980516910552978515625;-0.86039100000000001688960082901758141815662384033203125;-1.9658400000000000318323145620524883270263671875;0.1357530000000000125481847135233692824840545654296875;-0.0128716000000000004022115973612017114646732807159423828125;0.11394600000000000561417579092449159361422061920166015625;-1.0879600000000000381561449103173799812793731689453125;-1.0949199999999998933475353624089621007442474365234375;-1.9763200000000000766675611885148100554943084716796875;-0.6090109999999999690345475755748338997364044189453125;-0.7609230000000000160298441187478601932525634765625;-0.347119000000000010874856570808333344757556915283203125;-0.9725930000000000408277855967753566801548004150390625;-2.13919000000000014694023775518871843814849853515625;0.372145000000000003570477247194503434002399444580078125;-0.0297201999999999987023269198971320292912423610687255859375;-1.5436799999999999410960072054876945912837982177734375;-0.28163199999999999345590140364947728812694549560546875;-1.1462499999999999911182158029987476766109466552734375;1.252170000000000005258016244624741375446319580078125;-0.11740799999999999847499765337488497607409954071044921875;1.1355399999999999938182781988871283829212188720703125;-0.11044700000000000350564022255639429204165935516357421875;-1.9237699999999999800337491251411847770214080810546875;0.9183660000000000156461510414374060928821563720703125;-0.945281000000000037886138670728541910648345947265625;0.2480009999999999992237320611820905469357967376708984375;0.718034999999999978825826474349014461040496826171875;-1.285930000000000017479351299698464572429656982421875;-0.9102449999999999707966935602598823606967926025390625;0.5710699999999999665334371456992812454700469970703125;-1.149010000000000086828322309884242713451385498046875;-0.3951890000000000124913412946625612676143646240234375;-0.5170500000000000095923269327613525092601776123046875;0.46217900000000000648725517748971469700336456298828125;-1.0177099999999998924948840794968418776988983154296875;0.482476999999999989210408557482878677546977996826171875;-0.0224640000000000013280487820566122536547482013702392578125;0.84967800000000004434497213878785260021686553955078125;1.1124199999999999644018089384189806878566741943359375;-0.385116000000000013869794202037155628204345703125]
      mask Display: e = 0.06;c1 = [120 150 230]/255;c2 = ([120 150 230]+0)/383;c3 = ([120 150 230]+128)/383;patch([0 1 1 0],[0 0 1 1],c1);patch([0 1 1 1-e 1-e 0+e],[0 0 1 1-e 0+e 0+e],c2);patch([0 0 1 1-e 0+e 0+e],[0 1 1 1-e 1-e 0+e],c3);plot([0 1 1 0 0],[0 0 1 1 0]);disp('b');
  netsum : Sum  Inputs=2  SaturateOnIntegerOverflow=on  InputSameDT=on  OutDataTypeStr=Inherit: Same as first input
      mask Display: e = 0.06;c1 = [120 150 230]/255;c2 = ([120 150 230]+0)/383;c3 = ([120 150 230]+128)/383;patch([0 1 1 0],[0 0 1 1],c1);patch([0 1 1 1-e 1-e 0+e],[0 0 1 1-e 0+e 0+e],c2);patch([0 0 1 1-e 0+e 0+e],[0 1 1 1-e 1-e 0+e],c3);plot([0 1 1 0 0],[0 0 1 1 0]);disp('+');
  poslin : neural/Transfer Functions/poslin
      settings: SourceProductName=Deep Learning Toolbox  SourceProductBaseCode=NN
  "a{3}" : Outport  InitialOutput=[0;0;0;0;0;0;0;0;0;0;0;0;0;0;0;0;0;0;0;0;0;0;0;0;0;0;0;0;0;0;0;0;0;0;0;0;0;0;0;0;0;0;0;0;0;0;0;0;0;0]
      mask Display: e = 0.06;c1 = [120 230 180]/255;c2 = ([120 230 180]+0)/(256+128);c3 = ([120 230 180]+128)/(256+128);patch([0 1 1 0],[0 0 1 1],c1);patch([0 1 1 1-e 1-e 0+e],[0 0 1 1-e 0+e 0+e],c2);patch([0 0 1 1-e 0+e 0+e],[0 1 1 1-e 1-e 0+e],c3);plot([0 1 1 0 0],[0 0 1 1 0]);
  wires:
  poslin/1 -> "a{3}"/1
  netsum/1 -> poslin/1
  "b{3}"/1 -> netsum/2
  "LW{3,2}"/1("lz{3,2}") -> netsum/1
  Delays 1/1("pd{3,2}") -> "LW{3,2}"/1("ad{3,2}")
  "a{2} "/1 -> Delays 1/1("p{3}")

== system AcasXuClosedLoop_all_comp2017b/networks/Networks_m_4/AcasXu_3_4/Layer 4 ==
  "a{3} " : Inport  PortDimensions=50
      mask Display: e = 0.06;c1 = [120 230 180]/255;c2 = ([120 230 180]+0)/(256+128);c3 = ([120 230 180]+128)/(256+128);patch([0 1 1 0],[0 0 1 1],c1);patch([0 1 1 1-e 1-e 0+e],[0 0 1 1-e 0+e 0+e],c2);patch([0 0 1 1-e 0+e 0+e],[0 1 1 1-e 1-e 0+e],c3);plot([0 1 1 0 0],[0 0 1 1 0]);
  Delays 1 : Subsystem
      mask Display: e = 0.06;c1 = [120 150 230]/255;c2 = ([120 150 230]+0)/383;c3 = ([120 150 230]+128)/383;patch([0 1 1 0],[0 0 1 1],c1);patch([0 1 1 1-e 1-e 0+e],[0 0 1 1-e 0+e 0+e],c2);patch([0 0 1 1-e 0+e 0+e],[0 1 1 1-e 1-e 0+e],c3);plot([0 1 1 0 0],[0 0 1 1 0]);disp('0');
  "LW{4,3}" : Subsystem
      mask Display: e = 0.06;c1 = [120 150 230]/255;c2 = ([120 150 230]+0)/383;c3 = ([120 150 230]+128)/383;patch([0 1 1 0],[0 0 1 1],c1);patch([0 1 1 1-e 1-e 0+e],[0 0 1 1-e 0+e 0+e],c2);patch([0 0 1 1-e 0+e 0+e],[0 1 1 1-e 1-e 0+e],c3);plot([0 1 1 0 0],[0 0 1 1 0]);disp('W');
  "b{4}" : Constant  Value=[0.263714999999999977209341750494786538183689117431640625;-0.8912619999999999986783905114862136542797088623046875;-2.019579999999999930793137536966241896152496337890625;-0.19886999999999999122479721336276270449161529541015625;0.31599500000000002586375558166764676570892333984375;-0.037381699999999996875121865969049395062029361724853515625;-0.0236331000000000006344702541127844597212970256805419921875;-2.08434000000000008157030606525950133800506591796875;1.1567499999999999449329379785922355949878692626953125;-0.52585300000000001485744860474369488656520843505859375;-0.028188499999999998280042490250707487575709819793701171875;0.5284240000000000048174797484534792602062225341796875;0.354169000000000011585399306568433530628681182861328125;-0.75048999999999999044320020402665250003337860107421875;-0.57542700000000002180655656047747470438480377197265625;-1.2872099999999999653255144949071109294891357421875;0.059705500000000001625810597261079237796366214752197265625;-1.2876499999999999612754209010745398700237274169921875;-1.249989999999999934487959762918762862682342529296875;-0.70669999999999999484856516573927365243434906005859375;0.01419340000000000011348699757718350156210362911224365234375;-0.474057000000000006156852805361268110573291778564453125;0.97262700000000001931965698531712405383586883544921875;0.7932930000000000259063881458132527768611907958984375;0.15903400000000000868993765834602527320384979248046875;-0.89881800000000000583355586059042252600193023681640625;-1.65463000000000004519051799434237182140350341796875;-0.89407800000000003937117298846715129911899566650390625;0.36019699999999998940580780981690622866153717041015625;-0.106439000000000005830003146911622025072574615478515625;-2.345940000000000136282096718787215650081634521484375;-0.0275841999999999996917576794430715381167829036712646484375;-1.1812300000000000022026824808563105762004852294921875;-0.1153909999999999935749173118892940692603588104248046875;-0.57648500000000002518873998269555158913135528564453125;0.97118099999999996096988752469769679009914398193359375;0.65285599999999999187849653026205487549304962158203125;-0.0330174000000000023025137352306046523153781890869140625;-0.411272999999999999687361196265555918216705322265625;0.166754000000000013326229009180678986012935638427734375;-1.2423500000000000653699316899292171001434326171875;-1.3515900000000000691358081894577480852603912353515625;-1.9396500000000000962785406954935751855373382568359375;-0.93690200000000001256950099559617228806018829345703125;-0.7998110000000000496811480843462049961090087890625;-1.3160199999999999675992512493394315242767333984375;-1.0703300000000000036237679523765109479427337646484375;-2.339960000000000039932501749717630445957183837890625;-2.197080000000000143955958264996297657489776611328125;0.6624659999999999993036681189551018178462982177734375]
      mask Display: e = 0.06;c1 = [120 150 230]/255;c2 = ([120 150 230]+0)/383;c3 = ([120 150 230]+128)/383;patch([0 1 1 0],[0 0 1 1],c1);patch([0 1 1 1-e 1-e 0+e],[0 0 1 1-e 0+e 0+e],c2);patch([0 0 1 1-e 0+e 0+e],[0 1 1 1-e 1-e 0+e],c3);plot([0 1 1 0 0],[0 0 1 1 0]);disp('b');
  netsum : Sum  Inputs=2  SaturateOnIntegerOverflow=on  InputSameDT=on  OutDataTypeStr=Inherit: Same as first input
      mask Display: e = 0.06;c1 = [120 150 230]/255;c2 = ([120 150 230]+0)/383;c3 = ([120 150 230]+128)/383;patch([0 1 1 0],[0 0 1 1],c1);patch([0 1 1 1-e 1-e 0+e],[0 0 1 1-e 0+e 0+e],c2);patch([0 0 1 1-e 0+e 0+e],[0 1 1 1-e 1-e 0+e],c3);plot([0 1 1 0 0],[0 0 1 1 0]);disp('+');
  poslin : neural/Transfer Functions/poslin
      settings: SourceProductName=Deep Learning Toolbox  SourceProductBaseCode=NN
  "a{4}" : Outport  InitialOutput=[0;0;0;0;0;0;0;0;0;0;0;0;0;0;0;0;0;0;0;0;0;0;0;0;0;0;0;0;0;0;0;0;0;0;0;0;0;0;0;0;0;0;0;0;0;0;0;0;0;0]
      mask Display: e = 0.06;c1 = [120 230 180]/255;c2 = ([120 230 180]+0)/(256+128);c3 = ([120 230 180]+128)/(256+128);patch([0 1 1 0],[0 0 1 1],c1);patch([0 1 1 1-e 1-e 0+e],[0 0 1 1-e 0+e 0+e],c2);patch([0 0 1 1-e 0+e 0+e],[0 1 1 1-e 1-e 0+e],c3);plot([0 1 1 0 0],[0 0 1 1 0]);
  wires:
  poslin/1 -> "a{4}"/1
  netsum/1 -> poslin/1
  "b{4}"/1 -> netsum/2
  "LW{4,3}"/1("lz{4,3}") -> netsum/1
  Delays 1/1("pd{4,3}") -> "LW{4,3}"/1("ad{4,3}")
  "a{3} "/1 -> Delays 1/1("p{4}")
